simulink model "bee2_calib_corr"  (Simulink 7.1)
settings: Solver=ode45  StartTime=0.0  StopTime=100  RelTol=1e-3
undefined symbols (not defined in the file or in its folder): BEE2_usr
libraries: xbsIndex_r4 x2291, quant_xcorr_lib x1792, xps_library x56, xbsReplacements_r4 x32, casper_library_misc x2
(part 1 of 30)
settings Model: Creator=Administrator  LastModifiedBy=Jonathan Weintroub
settings DebuggingCC: SaveWithParameterizedLinksMsg=none  SignalResolutionControl=TryResolveAllWithWarning  MultiTaskDSMMsg=warning  MultiTaskCondExecSysMsg=none  StrictBusMsg=None
settings RTWCC: IncludeERTFirstTime=on
settings GRTTargetCC: TargetFcnLib=ansi_tfl_tmw.mat

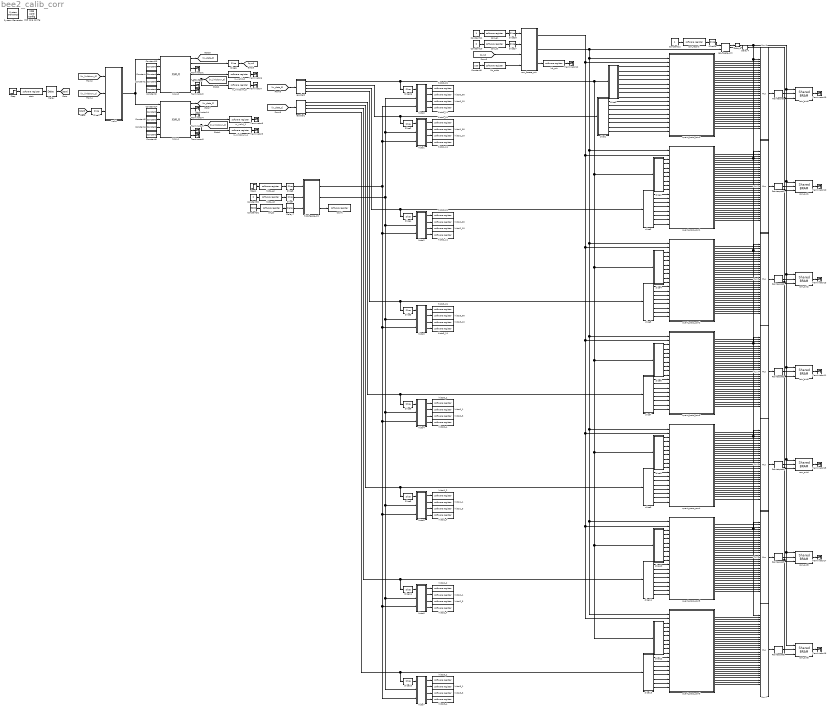
== system bee2_calib_corr ==
  " System Generator" : xbsIndex_r4/ System Generator  Tag=genX  SystemSampleTime=-1  xilinxfamily=virtex2p  part=xc2vp70  speed=-7  package=ff1704  synthesis_tool=XST  clock_wrapper=Clock Enables  directory=./bee2_calib_corr/sysgen  testbench=off  simulink_period=1  sysclk_period=10  dcm_input_clock_period=100  incr_netlist=off  trim_vbits=Everywhere in SubSystem  dbl_ovrd=According to Block Masks  core_generation=According to Block Masks  run_coregen=off  deprecated_control=off  eval_field=0  block_type=sysgen
      settings: block_version=10.1.3
  Constant : xbsIndex_r4/Constant  arith_type=Unsigned  const=0  n_bits=32  bin_pt=0  explicit_period=on  period=1  equ=P=C  opselect=C  inp2=PCIN>>17  opr=+  inp1=P  carry=CIN  dbl_ovrd=off  block_type=constant
  Constant1 : xbsIndex_r4/Constant  arith_type=Unsigned  const=0  n_bits=4  bin_pt=0  explicit_period=on  period=1  equ=P=C  opselect=C  inp2=PCIN>>17  opr=+  inp1=P  carry=CIN  dbl_ovrd=off  block_type=constant
  Constant10 : Constant  Value=0  OutDataType=sfix(16)  OutScaling=2^0
  Constant11 : Constant  OutDataType=sfix(16)  OutScaling=2^0
  Constant12 : Constant  OutDataType=sfix(16)  OutScaling=2^0
  Constant13 : xbsIndex_r4/Constant  arith_type=Boolean  const=0  n_bits=32  bin_pt=0  explicit_period=on  period=1  equ=P=C  opselect=C  inp2=PCIN>>17  opr=+  inp1=P  carry=CIN  dbl_ovrd=off  block_type=constant
  Constant14 : xbsIndex_r4/Constant  arith_type=Boolean  const=0  n_bits=32  bin_pt=0  explicit_period=on  period=1  equ=P=C  opselect=C  inp2=PCIN>>17  opr=+  inp1=P  carry=CIN  dbl_ovrd=off  block_type=constant
  Constant2 : xbsIndex_r4/Constant  arith_type=Boolean  const=0  n_bits=1  bin_pt=0  explicit_period=on  period=1  equ=P=C  opselect=C  inp2=PCIN>>17  opr=+  inp1=P  carry=CIN  dbl_ovrd=off  block_type=constant
  Constant3 : xbsIndex_r4/Constant  arith_type=Unsigned  const=0  n_bits=32  bin_pt=0  explicit_period=on  period=1  equ=P=C  opselect=C  inp2=PCIN>>17  opr=+  inp1=P  carry=CIN  dbl_ovrd=off  block_type=constant
  Constant31 : Constant  Value=5000  OutDataType=sfix(16)  OutScaling=2^0
  Constant32 : Constant  Value=0  OutDataType=sfix(16)  OutScaling=2^0
  Constant4 : xbsIndex_r4/Constant  arith_type=Unsigned  const=0  n_bits=4  bin_pt=0  explicit_period=on  period=1  equ=P=C  opselect=C  inp2=PCIN>>17  opr=+  inp1=P  carry=CIN  dbl_ovrd=off  block_type=constant
  Constant5 : xbsIndex_r4/Constant  arith_type=Boolean  const=0  n_bits=1  bin_pt=0  explicit_period=on  period=1  equ=P=C  opselect=C  inp2=PCIN>>17  opr=+  inp1=P  carry=CIN  dbl_ovrd=off  block_type=constant
  Constant8 : Constant  Value=100  OutDataType=sfix(16)  OutScaling=2^0
  Delay : xbsIndex_r4/Delay  en=off  latency=20  dbl_ovrd=off  reg_retiming=off  block_type=delay
      settings: xl_area=[0, 0, 0, 0, 0, 0, 0]
  Delay3 : xbsIndex_r4/Delay  en=off  latency=10  dbl_ovrd=off  reg_retiming=on  block_type=delay
      settings: xl_area=[0, 0, 0, 0, 0, 0, 0]
  Delay4 : xbsIndex_r4/Delay  en=off  latency=10  dbl_ovrd=off  reg_retiming=on  block_type=delay
      settings: xl_area=[0, 0, 0, 0, 0, 0, 0]
  Delay7 : xbsIndex_r4/Delay  en=off  latency=15  dbl_ovrd=off  reg_retiming=on  block_type=delay
      settings: xl_area=[0, 0, 0, 0, 0, 0, 0]
  Mux{1..7} (7 alike) : xbsIndex_r4/Mux  inputs=32  en=off  latency=0  precision=Full  arith_type="Signed  (2's comp)"  n_bits=8  bin_pt=2  quantization=Truncate  overflow=Wrap  dbl_ovrd=off  block_type=mux
      settings: xl_area=[0, 0, 0, 0, 0, 0, 0]
  Reinterpret{,1..6} (7 alike) : xbsIndex_r4/Reinterpret  force_arith_type=on  arith_type=Unsigned  force_bin_pt=on  bin_pt=0  block_type=reinterpret
      settings: infoedit="Changes signal type without altering the binary representation.   You can changed the signal between signed and unsigned, and relocate the binary point.<P><P>Hardware notes: In hardware this block costs nothing.<P><P>Example:  Suppose the input is 6 bits wide, signed, with 2 fractional bits, and the output is forced to unsigned with 0 fractional bits.  Then an input of -2.0 (1110.00 in binary 2's complement) becomes an output of 56 (111000 in binary)."  block_version=10.1.3
  Slice{1,6..8,27..29} (7 alike) : xbsIndex_r4/Slice  nbits=1  boolean_output=on  mode=Lower Bit Location + Width  bit1=0  base1=MSB of Input  bit0=0  base0=LSB of Input  dbl_ovrd=off  block_type=slice
      settings: infoedit="Extracts a given range of bits from each input sample and presents it at the output.  The output type is ordinarily unsigned with binary point at zero, but can be Boolean when the slice is one bit wide.<P><P>Hardware notes: In hardware this block costs nothing."  block_version=10.1.3
  Slice{2..5,9..11,26} (8 alike) : xbsIndex_r4/Slice  nbits=8  boolean_output=off  mode=Upper Bit Location + Width  bit1=0  base1=MSB of Input  bit0=0  base0=LSB of Input  dbl_ovrd=off  block_type=slice
      settings: infoedit="Extracts a given range of bits from each input sample and presents it at the output.  The output type is ordinarily unsigned with binary point at zero, but can be Boolean when the slice is one bit wide.<P><P>Hardware notes: In hardware this block costs nothing."  block_version=10.1.3
  Step : Step  Time=100  SampleTime=0
  Step1 : Step  Time=2000  SampleTime=0
  Terminator{,1..17,19} (19 alike) : Terminator
  XAUI_0 : xps_library/XAUI  Tag=xps:xaui  SystemSampleTime=-1  demux=1  port=BEE2_usr:0  open_phy=off  show_param=off  pre_emph=3  swing=800
      link override bee2_calib_corr_XAUI_0_rx_data: n_bits=64
      link override bee2_calib_corr_XAUI_0_rx_outofband: n_bits=8
      link override convert_tx_data: n_bits=64
      link override convert_tx_outofband: n_bits=8
  XAUI_1 : xps_library/XAUI  Tag=xps:xaui  SystemSampleTime=-1  demux=1  port=BEE2_usr:1  open_phy=off  show_param=off  pre_emph=3  swing=800
      link override bee2_calib_corr_XAUI_1_rx_data: n_bits=64
      link override bee2_calib_corr_XAUI_1_rx_outofband: n_bits=8
      link override convert_tx_data: n_bits=64
      link override convert_tx_outofband: n_bits=8
  XSG core config : xps_library/XSG core config  Tag=xps:xsg  SystemSampleTime=-1  hw_sys=BEE2_usr  ibob_linux=off  clk_src=sys_clk  iBOB_clk_src=sys_clk  BEE2_ctrl_clk_src=sys_clk  BEE2_usr_clk_src=sys_clk  ROACH_clk_src=sys_clk  CORR_clk_src=sys_clk  gpio_clk_io_group=iBOB:sma  gpio_clk_bit_index=0  clk_rate=100  sample_period=1  synthesis_tool=XST  mpc_type=powerpc405
  cnt_to : xps_library/software register  Tag=xps:sw_reg  SystemSampleTime=-1  io_dir=From Processor  arith_type=Unsigned  bitwidth=32  bin_pt=0  sample_period=1  gw_name=bee2_calib_corr_cnt_to_user_data_out
      link override bee2_calib_corr_cnt_to_user_data_out: arith_type=Unsigned  n_bits=32  bin_pt=0  period=1
  corr_en : xps_library/software register  Tag=xps:sw_reg  SystemSampleTime=-1  io_dir=From Processor  arith_type=Unsigned  bitwidth=32  bin_pt=0  sample_period=1  gw_name=bee2_calib_corr_corr_en_user_data_out
      link override bee2_calib_corr_corr_en_user_data_out: arith_type=Unsigned  n_bits=32  bin_pt=0  period=1
  corr_freeze_cnt : Subsystem
  corr_out0 : xps_library/Shared BRAM  Tag=xps:bram  SystemSampleTime=-1  arith_type="Signed  (2's comp)"  addr_width=11  data_width=32  data_bin_pt=0  init_vals=[1:2^11]  sample_rate=1
      link override bee2_calib_corr_corr_out0_data_out: arith_type="Signed  (2's comp)"
      link override convert_din: arith_type="Signed  (2's comp)"
      link override mem/sim_data_in: arith_type="Signed  (2's comp)"
  corr_out1 : xps_library/Shared BRAM  Tag=xps:bram  SystemSampleTime=-1  arith_type="Signed  (2's comp)"  addr_width=11  data_width=32  data_bin_pt=0  init_vals=[1:2^11]  sample_rate=1
      link override bee2_calib_corr_corr_out1_data_out: arith_type="Signed  (2's comp)"
      link override convert_din: arith_type="Signed  (2's comp)"
      link override mem/sim_data_in: arith_type="Signed  (2's comp)"
  corr_out2 : xps_library/Shared BRAM  Tag=xps:bram  SystemSampleTime=-1  arith_type="Signed  (2's comp)"  addr_width=11  data_width=32  data_bin_pt=0  init_vals=[1:2^11]  sample_rate=1
      link override bee2_calib_corr_corr_out2_data_out: arith_type="Signed  (2's comp)"
      link override convert_din: arith_type="Signed  (2's comp)"
      link override mem/sim_data_in: arith_type="Signed  (2's comp)"
  corr_out3 : xps_library/Shared BRAM  Tag=xps:bram  SystemSampleTime=-1  arith_type="Signed  (2's comp)"  addr_width=11  data_width=32  data_bin_pt=0  init_vals=[1:2^11]  sample_rate=1
      link override bee2_calib_corr_corr_out3_data_out: arith_type="Signed  (2's comp)"
      link override convert_din: arith_type="Signed  (2's comp)"
      link override mem/sim_data_in: arith_type="Signed  (2's comp)"
  corr_out4 : xps_library/Shared BRAM  Tag=xps:bram  SystemSampleTime=-1  arith_type="Signed  (2's comp)"  addr_width=11  data_width=32  data_bin_pt=0  init_vals=[1:2^11]  sample_rate=1
      link override bee2_calib_corr_corr_out4_data_out: arith_type="Signed  (2's comp)"
      link override convert_din: arith_type="Signed  (2's comp)"
      link override mem/sim_data_in: arith_type="Signed  (2's comp)"
  corr_out5 : xps_library/Shared BRAM  Tag=xps:bram  SystemSampleTime=-1  arith_type="Signed  (2's comp)"  addr_width=11  data_width=32  data_bin_pt=0  init_vals=[1:2^11]  sample_rate=1
      link override bee2_calib_corr_corr_out5_data_out: arith_type="Signed  (2's comp)"
      link override convert_din: arith_type="Signed  (2's comp)"
      link override mem/sim_data_in: arith_type="Signed  (2's comp)"
  corr_out6 : xps_library/Shared BRAM  Tag=xps:bram  SystemSampleTime=-1  arith_type="Signed  (2's comp)"  addr_width=11  data_width=32  data_bin_pt=0  init_vals=[1:2^11]  sample_rate=1
      link override bee2_calib_corr_corr_out6_data_out: arith_type="Signed  (2's comp)"
      link override convert_din: arith_type="Signed  (2's comp)"
      link override mem/sim_data_in: arith_type="Signed  (2's comp)"
  corr_record : xps_library/software register  Tag=xps:sw_reg  SystemSampleTime=-1  io_dir=From Processor  arith_type=Unsigned  bitwidth=32  bin_pt=0  sample_period=1  gw_name=bee2_calib_corr_corr_record_user_data_out
      link override bee2_calib_corr_corr_record_user_data_out: arith_type=Unsigned  n_bits=32  bin_pt=0  period=1
  corr_rst : xps_library/software register  Tag=xps:sw_reg  SystemSampleTime=-1  io_dir=From Processor  arith_type=Unsigned  bitwidth=32  bin_pt=0  sample_period=1  gw_name=bee2_calib_corr_corr_rst_user_data_out
      link override bee2_calib_corr_corr_rst_user_data_out: arith_type=Unsigned  n_bits=32  bin_pt=0  period=1
  count : xps_library/software register  Tag=xps:sw_reg  SystemSampleTime=-1  io_dir=To Processor  arith_type=Unsigned  bitwidth=32  bin_pt=0  sample_period=1  gw_name=bee2_calib_corr_count_user_data_in
      link override convert: arith_type=Unsigned  n_bits=32  bin_pt=0
  hb_cnt : xps_library/software register  Tag=xps:sw_reg  SystemSampleTime=-1  io_dir=To Processor  arith_type=Unsigned  bitwidth=32  bin_pt=0  sample_period=1  gw_name=bee2_calib_corr_hb_cnt_user_data_in
      link override convert: arith_type=Unsigned  n_bits=32  bin_pt=0
  hb_cntto : xps_library/software register  Tag=xps:sw_reg  SystemSampleTime=-1  io_dir=From Processor  arith_type=Unsigned  bitwidth=32  bin_pt=0  sample_period=1  gw_name=bee2_calib_corr_hb_cntto_user_data_out
      link override bee2_calib_corr_hb_cntto_user_data_out: arith_type=Unsigned  n_bits=32  bin_pt=0  period=1
  hist_freeze_cnt : Subsystem
  histo0 : Subsystem
  histo0_00 : xps_library/software register  Tag=xps:sw_reg  SystemSampleTime=-1  io_dir=To Processor  arith_type=Unsigned  bitwidth=32  bin_pt=0  sample_period=1  gw_name=bee2_calib_corr_histo0_00_user_data_in
      link override convert: arith_type=Unsigned  n_bits=32  bin_pt=0
  histo0_01 : xps_library/software register  Tag=xps:sw_reg  SystemSampleTime=-1  io_dir=To Processor  arith_type=Unsigned  bitwidth=32  bin_pt=0  sample_period=1  gw_name=bee2_calib_corr_histo0_01_user_data_in
      link override convert: arith_type=Unsigned  n_bits=32  bin_pt=0
  histo0_1 : xps_library/software register  Tag=xps:sw_reg  SystemSampleTime=-1  io_dir=To Processor  arith_type=Unsigned  bitwidth=32  bin_pt=0  sample_period=1  gw_name=bee2_calib_corr_histo0_1_user_data_in
      link override convert: arith_type=Unsigned  n_bits=32  bin_pt=0
  histo0_10 : xps_library/software register  Tag=xps:sw_reg  SystemSampleTime=-1  io_dir=To Processor  arith_type=Unsigned  bitwidth=32  bin_pt=0  sample_period=1  gw_name=bee2_calib_corr_histo0_10_user_data_in
      link override convert: arith_type=Unsigned  n_bits=32  bin_pt=0
  histo0_11 : xps_library/software register  Tag=xps:sw_reg  SystemSampleTime=-1  io_dir=To Processor  arith_type=Unsigned  bitwidth=32  bin_pt=0  sample_period=1  gw_name=bee2_calib_corr_histo0_11_user_data_in
      link override convert: arith_type=Unsigned  n_bits=32  bin_pt=0
  histo0_2 : xps_library/software register  Tag=xps:sw_reg  SystemSampleTime=-1  io_dir=To Processor  arith_type=Unsigned  bitwidth=32  bin_pt=0  sample_period=1  gw_name=bee2_calib_corr_histo0_2_user_data_in
      link override convert: arith_type=Unsigned  n_bits=32  bin_pt=0
  histo0_3 : xps_library/software register  Tag=xps:sw_reg  SystemSampleTime=-1  io_dir=To Processor  arith_type=Unsigned  bitwidth=32  bin_pt=0  sample_period=1  gw_name=bee2_calib_corr_histo0_3_user_data_in
      link override convert: arith_type=Unsigned  n_bits=32  bin_pt=0
  histo0_4 : xps_library/software register  Tag=xps:sw_reg  SystemSampleTime=-1  io_dir=To Processor  arith_type=Unsigned  bitwidth=32  bin_pt=0  sample_period=1  gw_name=bee2_calib_corr_histo0_4_user_data_in
      link override convert: arith_type=Unsigned  n_bits=32  bin_pt=0
  histo1 : Subsystem
  histo1_00 : xps_library/software register  Tag=xps:sw_reg  SystemSampleTime=-1  io_dir=To Processor  arith_type=Unsigned  bitwidth=32  bin_pt=0  sample_period=1  gw_name=bee2_calib_corr_histo1_00_user_data_in
      link override convert: arith_type=Unsigned  n_bits=32  bin_pt=0
  histo1_01 : xps_library/software register  Tag=xps:sw_reg  SystemSampleTime=-1  io_dir=To Processor  arith_type=Unsigned  bitwidth=32  bin_pt=0  sample_period=1  gw_name=bee2_calib_corr_histo1_01_user_data_in
      link override convert: arith_type=Unsigned  n_bits=32  bin_pt=0
  histo1_1 : xps_library/software register  Tag=xps:sw_reg  SystemSampleTime=-1  io_dir=To Processor  arith_type=Unsigned  bitwidth=32  bin_pt=0  sample_period=1  gw_name=bee2_calib_corr_histo1_1_user_data_in
      link override convert: arith_type=Unsigned  n_bits=32  bin_pt=0
  histo1_10 : xps_library/software register  Tag=xps:sw_reg  SystemSampleTime=-1  io_dir=To Processor  arith_type=Unsigned  bitwidth=32  bin_pt=0  sample_period=1  gw_name=bee2_calib_corr_histo1_10_user_data_in
      link override convert: arith_type=Unsigned  n_bits=32  bin_pt=0
  histo1_11 : xps_library/software register  Tag=xps:sw_reg  SystemSampleTime=-1  io_dir=To Processor  arith_type=Unsigned  bitwidth=32  bin_pt=0  sample_period=1  gw_name=bee2_calib_corr_histo1_11_user_data_in
      link override convert: arith_type=Unsigned  n_bits=32  bin_pt=0
  histo1_2 : xps_library/software register  Tag=xps:sw_reg  SystemSampleTime=-1  io_dir=To Processor  arith_type=Unsigned  bitwidth=32  bin_pt=0  sample_period=1  gw_name=bee2_calib_corr_histo1_2_user_data_in
      link override convert: arith_type=Unsigned  n_bits=32  bin_pt=0
  histo1_3 : xps_library/software register  Tag=xps:sw_reg  SystemSampleTime=-1  io_dir=To Processor  arith_type=Unsigned  bitwidth=32  bin_pt=0  sample_period=1  gw_name=bee2_calib_corr_histo1_3_user_data_in
      link override convert: arith_type=Unsigned  n_bits=32  bin_pt=0
  histo1_4 : xps_library/software register  Tag=xps:sw_reg  SystemSampleTime=-1  io_dir=To Processor  arith_type=Unsigned  bitwidth=32  bin_pt=0  sample_period=1  gw_name=bee2_calib_corr_histo1_4_user_data_in
      link override convert: arith_type=Unsigned  n_bits=32  bin_pt=0
  histo2 : Subsystem
  histo2_00 : xps_library/software register  Tag=xps:sw_reg  SystemSampleTime=-1  io_dir=To Processor  arith_type=Unsigned  bitwidth=32  bin_pt=0  sample_period=1  gw_name=bee2_calib_corr_histo2_00_user_data_in
      link override convert: arith_type=Unsigned  n_bits=32  bin_pt=0
  histo2_01 : xps_library/software register  Tag=xps:sw_reg  SystemSampleTime=-1  io_dir=To Processor  arith_type=Unsigned  bitwidth=32  bin_pt=0  sample_period=1  gw_name=bee2_calib_corr_histo2_01_user_data_in
      link override convert: arith_type=Unsigned  n_bits=32  bin_pt=0
  histo2_1 : xps_library/software register  Tag=xps:sw_reg  SystemSampleTime=-1  io_dir=To Processor  arith_type=Unsigned  bitwidth=32  bin_pt=0  sample_period=1  gw_name=bee2_calib_corr_histo2_1_user_data_in
      link override convert: arith_type=Unsigned  n_bits=32  bin_pt=0
  histo2_10 : xps_library/software register  Tag=xps:sw_reg  SystemSampleTime=-1  io_dir=To Processor  arith_type=Unsigned  bitwidth=32  bin_pt=0  sample_period=1  gw_name=bee2_calib_corr_histo2_10_user_data_in
      link override convert: arith_type=Unsigned  n_bits=32  bin_pt=0
  histo2_11 : xps_library/software register  Tag=xps:sw_reg  SystemSampleTime=-1  io_dir=To Processor  arith_type=Unsigned  bitwidth=32  bin_pt=0  sample_period=1  gw_name=bee2_calib_corr_histo2_11_user_data_in
      link override convert: arith_type=Unsigned  n_bits=32  bin_pt=0
  histo2_2 : xps_library/software register  Tag=xps:sw_reg  SystemSampleTime=-1  io_dir=To Processor  arith_type=Unsigned  bitwidth=32  bin_pt=0  sample_period=1  gw_name=bee2_calib_corr_histo2_2_user_data_in
      link override convert: arith_type=Unsigned  n_bits=32  bin_pt=0
  histo2_3 : xps_library/software register  Tag=xps:sw_reg  SystemSampleTime=-1  io_dir=To Processor  arith_type=Unsigned  bitwidth=32  bin_pt=0  sample_period=1  gw_name=bee2_calib_corr_histo2_3_user_data_in
      link override convert: arith_type=Unsigned  n_bits=32  bin_pt=0
  histo2_4 : xps_library/software register  Tag=xps:sw_reg  SystemSampleTime=-1  io_dir=To Processor  arith_type=Unsigned  bitwidth=32  bin_pt=0  sample_period=1  gw_name=bee2_calib_corr_histo2_4_user_data_in
      link override convert: arith_type=Unsigned  n_bits=32  bin_pt=0
  histo3 : Subsystem
  histo3_00 : xps_library/software register  Tag=xps:sw_reg  SystemSampleTime=-1  io_dir=To Processor  arith_type=Unsigned  bitwidth=32  bin_pt=0  sample_period=1  gw_name=bee2_calib_corr_histo3_00_user_data_in
      link override convert: arith_type=Unsigned  n_bits=32  bin_pt=0
  histo3_01 : xps_library/software register  Tag=xps:sw_reg  SystemSampleTime=-1  io_dir=To Processor  arith_type=Unsigned  bitwidth=32  bin_pt=0  sample_period=1  gw_name=bee2_calib_corr_histo3_01_user_data_in
      link override convert: arith_type=Unsigned  n_bits=32  bin_pt=0
  histo3_1 : xps_library/software register  Tag=xps:sw_reg  SystemSampleTime=-1  io_dir=To Processor  arith_type=Unsigned  bitwidth=32  bin_pt=0  sample_period=1  gw_name=bee2_calib_corr_histo3_1_user_data_in
      link override convert: arith_type=Unsigned  n_bits=32  bin_pt=0
  histo3_10 : xps_library/software register  Tag=xps:sw_reg  SystemSampleTime=-1  io_dir=To Processor  arith_type=Unsigned  bitwidth=32  bin_pt=0  sample_period=1  gw_name=bee2_calib_corr_histo3_10_user_data_in
      link override convert: arith_type=Unsigned  n_bits=32  bin_pt=0
  histo3_11 : xps_library/software register  Tag=xps:sw_reg  SystemSampleTime=-1  io_dir=To Processor  arith_type=Unsigned  bitwidth=32  bin_pt=0  sample_period=1  gw_name=bee2_calib_corr_histo3_11_user_data_in
      link override convert: arith_type=Unsigned  n_bits=32  bin_pt=0
  histo3_2 : xps_library/software register  Tag=xps:sw_reg  SystemSampleTime=-1  io_dir=To Processor  arith_type=Unsigned  bitwidth=32  bin_pt=0  sample_period=1  gw_name=bee2_calib_corr_histo3_2_user_data_in
      link override convert: arith_type=Unsigned  n_bits=32  bin_pt=0
  histo3_3 : xps_library/software register  Tag=xps:sw_reg  SystemSampleTime=-1  io_dir=To Processor  arith_type=Unsigned  bitwidth=32  bin_pt=0  sample_period=1  gw_name=bee2_calib_corr_histo3_3_user_data_in
      link override convert: arith_type=Unsigned  n_bits=32  bin_pt=0
  histo3_4 : xps_library/software register  Tag=xps:sw_reg  SystemSampleTime=-1  io_dir=To Processor  arith_type=Unsigned  bitwidth=32  bin_pt=0  sample_period=1  gw_name=bee2_calib_corr_histo3_4_user_data_in
      link override convert: arith_type=Unsigned  n_bits=32  bin_pt=0
  histo4 : Subsystem
  histo5 : Subsystem
  histo6 : Subsystem
  histo7 : Subsystem
  histo_en : xps_library/software register  Tag=xps:sw_reg  SystemSampleTime=-1  io_dir=From Processor  arith_type=Unsigned  bitwidth=32  bin_pt=0  sample_period=1  gw_name=bee2_calib_corr_histo_en_user_data_out
      link override bee2_calib_corr_histo_en_user_data_out: arith_type=Unsigned  n_bits=32  bin_pt=0  period=1
  histo_rst : xps_library/software register  Tag=xps:sw_reg  SystemSampleTime=-1  io_dir=From Processor  arith_type=Unsigned  bitwidth=32  bin_pt=0  sample_period=1  gw_name=bee2_calib_corr_histo_rst_user_data_out
      link override bee2_calib_corr_histo_rst_user_data_out: arith_type=Unsigned  n_bits=32  bin_pt=0  period=1
  quant_cross_corr0 : Subsystem  AncestorBlock=quant_xcorr_lib/quant_cross_corr  mask(quant_cross_corr){ants=2, demuxr2=3, bits=2, lags=4, range=positive and negative lags}
      mask prompts: ants "Antennas:"; demuxr2 "Demux (2^?):"; bits "Bitwidth:"; lags "Lags (in multiples of demux):"
      mask init:
      | quant_cross_corr_init(gcb,...
      |     'ants', ants,...
      |     'demuxr2', demuxr2,...
      |     'bits', bits,...
      |     'lags', lags,...
      |     'range', range);
      settings: AttributesFormatString=antennas=2, demux=8, lags=32
      mask StyleString: edit,edit,edit,edit,popup(positive and negative lags|positive lags only)
  quant_cross_corr1 : Subsystem  AncestorBlock=quant_xcorr_lib/quant_cross_corr  mask(quant_cross_corr){ants=2, demuxr2=3, bits=2, lags=4, range=positive and negative lags}
      mask prompts: ants "Antennas:"; demuxr2 "Demux (2^?):"; bits "Bitwidth:"; lags "Lags (in multiples of demux):"
      mask init:
      | quant_cross_corr_init(gcb,...
      |     'ants', ants,...
      |     'demuxr2', demuxr2,...
      |     'bits', bits,...
      |     'lags', lags,...
      |     'range', range);
      settings: AttributesFormatString=antennas=2, demux=8, lags=32
      mask StyleString: edit,edit,edit,edit,popup(positive and negative lags|positive lags only)
  quant_cross_corr2 : Subsystem  AncestorBlock=quant_xcorr_lib/quant_cross_corr  mask(quant_cross_corr){ants=2, demuxr2=3, bits=2, lags=4, range=positive and negative lags}
      mask prompts: ants "Antennas:"; demuxr2 "Demux (2^?):"; bits "Bitwidth:"; lags "Lags (in multiples of demux):"
      mask init:
      | quant_cross_corr_init(gcb,...
      |     'ants', ants,...
      |     'demuxr2', demuxr2,...
      |     'bits', bits,...
      |     'lags', lags,...
      |     'range', range);
      settings: AttributesFormatString=antennas=2, demux=8, lags=32
      mask StyleString: edit,edit,edit,edit,popup(positive and negative lags|positive lags only)
  quant_cross_corr3 : Subsystem  AncestorBlock=quant_xcorr_lib/quant_cross_corr  mask(quant_cross_corr){ants=2, demuxr2=3, bits=2, lags=4, range=positive and negative lags}
      mask prompts: ants "Antennas:"; demuxr2 "Demux (2^?):"; bits "Bitwidth:"; lags "Lags (in multiples of demux):"
      mask init:
      | quant_cross_corr_init(gcb,...
      |     'ants', ants,...
      |     'demuxr2', demuxr2,...
      |     'bits', bits,...
      |     'lags', lags,...
      |     'range', range);
      settings: AttributesFormatString=antennas=2, demux=8, lags=32
      mask StyleString: edit,edit,edit,edit,popup(positive and negative lags|positive lags only)
  quant_cross_corr4 : Subsystem  AncestorBlock=quant_xcorr_lib/quant_cross_corr  mask(quant_cross_corr){ants=2, demuxr2=3, bits=2, lags=4, range=positive and negative lags}
      mask prompts: ants "Antennas:"; demuxr2 "Demux (2^?):"; bits "Bitwidth:"; lags "Lags (in multiples of demux):"
      mask init:
      | quant_cross_corr_init(gcb,...
      |     'ants', ants,...
      |     'demuxr2', demuxr2,...
      |     'bits', bits,...
      |     'lags', lags,...
      |     'range', range);
      settings: AttributesFormatString=antennas=2, demux=8, lags=32
      mask StyleString: edit,edit,edit,edit,popup(positive and negative lags|positive lags only)
  quant_cross_corr5 : Subsystem  AncestorBlock=quant_xcorr_lib/quant_cross_corr  mask(quant_cross_corr){ants=2, demuxr2=3, bits=2, lags=4, range=positive and negative lags}
      mask prompts: ants "Antennas:"; demuxr2 "Demux (2^?):"; bits "Bitwidth:"; lags "Lags (in multiples of demux):"
      mask init:
      | quant_cross_corr_init(gcb,...
      |     'ants', ants,...
      |     'demuxr2', demuxr2,...
      |     'bits', bits,...
      |     'lags', lags,...
      |     'range', range);
      settings: AttributesFormatString=antennas=2, demux=8, lags=32
      mask StyleString: edit,edit,edit,edit,popup(positive and negative lags|positive lags only)
  quant_cross_corr6 : Subsystem  AncestorBlock=quant_xcorr_lib/quant_cross_corr  mask(quant_cross_corr){ants=2, demuxr2=3, bits=2, lags=4, range=positive and negative lags}
      mask prompts: ants "Antennas:"; demuxr2 "Demux (2^?):"; bits "Bitwidth:"; lags "Lags (in multiples of demux):"
      mask init:
      | quant_cross_corr_init(gcb,...
      |     'ants', ants,...
      |     'demuxr2', demuxr2,...
      |     'bits', bits,...
      |     'lags', lags,...
      |     'range', range);
      settings: AttributesFormatString=antennas=2, demux=8, lags=32
      mask StyleString: edit,edit,edit,edit,popup(positive and negative lags|positive lags only)
  rec_freeze_cnt : casper_library_misc/freeze_cntr  SystemSampleTime=-1  CounterBits=5
  reorder0 : Subsystem
  reorder1 : Subsystem
  rx_linkdown_0 : xps_library/software register  Tag=xps:sw_reg  SystemSampleTime=-1  io_dir=To Processor  arith_type=Unsigned  bitwidth=32  bin_pt=0  sample_period=1  gw_name=bee2_calib_corr_rx_linkdown_0_user_data_in
      link override convert: arith_type=Unsigned  n_bits=32  bin_pt=0
  rx_linkdown_1 : xps_library/software register  Tag=xps:sw_reg  SystemSampleTime=-1  io_dir=To Processor  arith_type=Unsigned  bitwidth=32  bin_pt=0  sample_period=1  gw_name=bee2_calib_corr_rx_linkdown_1_user_data_in
      link override convert: arith_type=Unsigned  n_bits=32  bin_pt=0
  rx_valid_0 : xps_library/software register  Tag=xps:sw_reg  SystemSampleTime=-1  io_dir=To Processor  arith_type=Unsigned  bitwidth=32  bin_pt=0  sample_period=1  gw_name=bee2_calib_corr_rx_valid_0_user_data_in
      link override convert: arith_type=Unsigned  n_bits=32  bin_pt=0
  rx_valid_1 : xps_library/software register  Tag=xps:sw_reg  SystemSampleTime=-1  io_dir=To Processor  arith_type=Unsigned  bitwidth=32  bin_pt=0  sample_period=1  gw_name=bee2_calib_corr_rx_valid_1_user_data_in
      link override convert: arith_type=Unsigned  n_bits=32  bin_pt=0
  slice0 : Subsystem
  slice1 : Subsystem
  slice10 : Subsystem
  slice11 : Subsystem
  slice12 : Subsystem
  slice13 : Subsystem
  slice2 : Subsystem
  slice3 : Subsystem
  slice4 : Subsystem
  slice5 : Subsystem
  slice6 : Subsystem
  slice7 : Subsystem
  slice8 : Subsystem
  slice9 : Subsystem
  start : xps_library/software register  Tag=xps:sw_reg  SystemSampleTime=-1  io_dir=From Processor  arith_type=Unsigned  bitwidth=32  bin_pt=0  sample_period=1  gw_name=bee2_calib_corr_start_user_data_out
      link override bee2_calib_corr_start_user_data_out: arith_type=Unsigned  n_bits=32  bin_pt=0  period=1
  valid : Subsystem
  wires:
  Step/1 -> start/1
  start/1 -> Delay/1
  Delay/1 -> [start]
  Constant/1 -> XAUI_0/3
  Constant1/1 -> XAUI_0/4
  Constant2/1 -> XAUI_0/5
  Constant3/1 -> XAUI_1/3
  Constant4/1 -> XAUI_1/4
  Constant5/1 -> XAUI_1/5
  XAUI_0/3 -> Terminator1/1
  XAUI_0/6 -> Terminator/1
  XAUI_0/7 -> Terminator2/1
  XAUI_1/3 -> Terminator4/1
  XAUI_1/6 -> Terminator3/1
  XAUI_1/7 -> Terminator5/1
  XAUI_0/4 -> rx_valid_0/1
  XAUI_0/5 -> rx_linkdown_0/1, [rx_linkdown_0]
  rx_valid_0/1 -> Terminator6/1
  rx_linkdown_0/1 -> Terminator7/1
  XAUI_1/4 -> rx_valid_1/1
  XAUI_1/5 -> [rx_linkdown_1], rx_linkdown_1/1
  rx_valid_1/1 -> Terminator8/1
  rx_linkdown_1/1 -> Terminator9/1
  [rx_linkdown_0] -> valid/1(ld0)
  [rx_linkdown_1] -> valid/2(ld1)
  XAUI_0/1 -> [rx_data_0]
  XAUI_1/1 -> [rx_data_1]
  [start] -> Slice1/1
  Slice1/1 -> valid/3(align)
  corr_freeze_cnt/2(rst_out) -> rec_freeze_cnt/2, quant_cross_corr0/1(rst), quant_cross_corr1/1(rst), quant_cross_corr2/1(rst), quant_cross_corr3/1(rst), quant_cross_corr6/1(rst), quant_cross_corr5/1(rst), quant_cross_corr4/1(rst)
  corr_freeze_cnt/1(en_out) -> quant_cross_corr0/2(en), quant_cross_corr1/2(en), quant_cross_corr2/2(en), quant_cross_corr3/2(en), quant_cross_corr6/2(en), quant_cross_corr5/2(en), quant_cross_corr4/2(en)
  corr_record/1 -> Slice29/1
  Constant12/1 -> corr_record/1
  Slice29/1 -> rec_freeze_cnt/1
  rec_freeze_cnt/1 -> Delay3/1
  Mux1/1 -> Reinterpret/1
  rec_freeze_cnt/2 -> Delay4/1
  corr_out0/1 -> Terminator19/1
  Reinterpret/1 -> corr_out0/2
  quant_cross_corr0/1(out-16) -> Mux1/2
  quant_cross_corr0/2(out-15) -> Mux1/3
  quant_cross_corr0/3(out-14) -> Mux1/4
  quant_cross_corr0/4(out-13) -> Mux1/5
  quant_cross_corr0/5(out-12) -> Mux1/6
  quant_cross_corr0/6(out-11) -> Mux1/7
  quant_cross_corr0/7(out-10) -> Mux1/8
  quant_cross_corr0/8(out-9) -> Mux1/9
  quant_cross_corr0/9(out-8) -> Mux1/10
  quant_cross_corr0/10(out-7) -> Mux1/11
  quant_cross_corr0/11(out-6) -> Mux1/12
  quant_cross_corr0/12(out-5) -> Mux1/13
  quant_cross_corr0/13(out-4) -> Mux1/14
  quant_cross_corr0/14(out-3) -> Mux1/15
  quant_cross_corr0/15(out-2) -> Mux1/16
  quant_cross_corr0/16(out-1) -> Mux1/17
  quant_cross_corr0/17(out0) -> Mux1/18
  quant_cross_corr0/18(out1) -> Mux1/19
  quant_cross_corr0/19(out2) -> Mux1/20
  quant_cross_corr0/20(out3) -> Mux1/21
  quant_cross_corr0/21(out4) -> Mux1/22
  quant_cross_corr0/22(out5) -> Mux1/23
  quant_cross_corr0/23(out6) -> Mux1/24
  quant_cross_corr0/24(out7) -> Mux1/25
  quant_cross_corr0/25(out8) -> Mux1/26
  quant_cross_corr0/26(out9) -> Mux1/27
  quant_cross_corr0/27(out10) -> Mux1/28
  quant_cross_corr0/28(out11) -> Mux1/29
  quant_cross_corr0/29(out12) -> Mux1/30
  quant_cross_corr0/30(out13) -> Mux1/31
  quant_cross_corr0/31(out14) -> Mux1/32
  quant_cross_corr0/32(out15) -> Mux1/33
  XAUI_0/2 -> Slice6/1
  XAUI_1/2 -> Terminator14/1
  valid/1(get) -> XAUI_0/1, XAUI_1/1
  Constant13/1 -> XAUI_0/2
  Constant14/1 -> XAUI_1/2
  [rx_data_0] -> reorder0/1(in)
  [rx_data_1] -> reorder1/1(in)
  slice0/1(out0) -> quant_cross_corr0/3(in1_16)
  slice0/2(out1) -> quant_cross_corr0/4(in1_17)
  slice0/3(out2) -> quant_cross_corr0/5(in1_18)
  slice0/4(out3) -> quant_cross_corr0/6(in1_19)
  slice0/5(out4) -> quant_cross_corr0/7(in1_20)
  slice0/6(out5) -> quant_cross_corr0/8(in1_21)
  slice0/7(out6) -> quant_cross_corr0/9(in1_22)
  slice0/8(out7) -> quant_cross_corr0/10(in1_23)
  slice1/1(out0) -> quant_cross_corr0/11(in2_16)
  slice1/2(out1) -> quant_cross_corr0/12(in2_17)
  slice1/3(out2) -> quant_cross_corr0/13(in2_18)
  slice1/4(out3) -> quant_cross_corr0/14(in2_19)
  slice1/5(out4) -> quant_cross_corr0/15(in2_20)
  slice1/6(out5) -> quant_cross_corr0/16(in2_21)
  slice1/7(out6) -> quant_cross_corr0/17(in2_22)
  slice1/8(out7) -> quant_cross_corr0/18(in2_23)
  Mux2/1 -> Reinterpret1/1
  corr_out1/1 -> Terminator10/1
  Reinterpret1/1 -> corr_out1/2
  quant_cross_corr1/8(out-9) -> Mux2/9
  quant_cross_corr1/9(out-8) -> Mux2/10
  quant_cross_corr1/10(out-7) -> Mux2/11
  quant_cross_corr1/11(out-6) -> Mux2/12
  quant_cross_corr1/12(out-5) -> Mux2/13
  quant_cross_corr1/13(out-4) -> Mux2/14
  quant_cross_corr1/14(out-3) -> Mux2/15
  quant_cross_corr1/15(out-2) -> Mux2/16
  quant_cross_corr1/16(out-1) -> Mux2/17
  quant_cross_corr1/17(out0) -> Mux2/18
  quant_cross_corr1/18(out1) -> Mux2/19
  quant_cross_corr1/19(out2) -> Mux2/20
  quant_cross_corr1/20(out3) -> Mux2/21
  quant_cross_corr1/21(out4) -> Mux2/22
  quant_cross_corr1/22(out5) -> Mux2/23
  quant_cross_corr1/23(out6) -> Mux2/24
  quant_cross_corr1/24(out7) -> Mux2/25
  quant_cross_corr1/25(out8) -> Mux2/26
  quant_cross_corr1/26(out9) -> Mux2/27
  quant_cross_corr1/27(out10) -> Mux2/28
  quant_cross_corr1/28(out11) -> Mux2/29
  quant_cross_corr1/29(out12) -> Mux2/30
  quant_cross_corr1/30(out13) -> Mux2/31
  quant_cross_corr1/31(out14) -> Mux2/32
  quant_cross_corr1/32(out15) -> Mux2/33
  slice2/3(out2) -> quant_cross_corr1/5(in1_18)
  slice2/4(out3) -> quant_cross_corr1/6(in1_19)
  slice2/5(out4) -> quant_cross_corr1/7(in1_20)
  slice2/6(out5) -> quant_cross_corr1/8(in1_21)
  slice2/7(out6) -> quant_cross_corr1/9(in1_22)
  slice2/8(out7) -> quant_cross_corr1/10(in1_23)
  slice3/1(out0) -> quant_cross_corr1/11(in2_16)
  slice3/2(out1) -> quant_cross_corr1/12(in2_17)
  slice3/3(out2) -> quant_cross_corr1/13(in2_18)
  slice3/4(out3) -> quant_cross_corr1/14(in2_19)
  slice3/5(out4) -> quant_cross_corr1/15(in2_20)
  slice3/6(out5) -> quant_cross_corr1/16(in2_21)
  slice3/7(out6) -> quant_cross_corr1/17(in2_22)
  slice3/8(out7) -> quant_cross_corr1/18(in2_23)
  quant_cross_corr1/1(out-16) -> Mux2/2
  quant_cross_corr1/2(out-15) -> Mux2/3
  quant_cross_corr1/3(out-14) -> Mux2/4
  quant_cross_corr1/4(out-13) -> Mux2/5
  quant_cross_corr1/5(out-12) -> Mux2/6
  quant_cross_corr1/6(out-11) -> Mux2/7
  quant_cross_corr1/7(out-10) -> Mux2/8
  reorder0/1(out0) -> Slice26/1, slice2/1(in), slice6/1(in), slice8/1(in), slice12/1(in), slice10/1(in), slice4/1(in), slice0/1(in)
  reorder0/2(out1) -> slice1/1(in), Slice2/1
  reorder0/3(out2) -> slice3/1(in), Slice4/1
  Mux3/1 -> Reinterpret2/1
  corr_out2/1 -> Terminator13/1
  Reinterpret2/1 -> corr_out2/2
  quant_cross_corr2/1(out-16) -> Mux3/2
  quant_cross_corr2/2(out-15) -> Mux3/3
  quant_cross_corr2/3(out-14) -> Mux3/4
  quant_cross_corr2/4(out-13) -> Mux3/5
  quant_cross_corr2/5(out-12) -> Mux3/6
  quant_cross_corr2/6(out-11) -> Mux3/7
  quant_cross_corr2/7(out-10) -> Mux3/8
  quant_cross_corr2/8(out-9) -> Mux3/9
  quant_cross_corr2/9(out-8) -> Mux3/10
  quant_cross_corr2/10(out-7) -> Mux3/11
  quant_cross_corr2/11(out-6) -> Mux3/12
  quant_cross_corr2/12(out-5) -> Mux3/13
  quant_cross_corr2/13(out-4) -> Mux3/14
  quant_cross_corr2/14(out-3) -> Mux3/15
  quant_cross_corr2/15(out-2) -> Mux3/16
  quant_cross_corr2/16(out-1) -> Mux3/17
  quant_cross_corr2/17(out0) -> Mux3/18
  quant_cross_corr2/18(out1) -> Mux3/19
  quant_cross_corr2/19(out2) -> Mux3/20
  quant_cross_corr2/20(out3) -> Mux3/21
  quant_cross_corr2/21(out4) -> Mux3/22
  quant_cross_corr2/22(out5) -> Mux3/23
  quant_cross_corr2/23(out6) -> Mux3/24
  quant_cross_corr2/24(out7) -> Mux3/25
  quant_cross_corr2/25(out8) -> Mux3/26
  quant_cross_corr2/26(out9) -> Mux3/27
  quant_cross_corr2/27(out10) -> Mux3/28
  quant_cross_corr2/28(out11) -> Mux3/29
  quant_cross_corr2/29(out12) -> Mux3/30
  quant_cross_corr2/30(out13) -> Mux3/31
  quant_cross_corr2/31(out14) -> Mux3/32
  quant_cross_corr2/32(out15) -> Mux3/33
  slice4/6(out5) -> quant_cross_corr2/8(in1_21)
  slice4/7(out6) -> quant_cross_corr2/9(in1_22)
  slice4/8(out7) -> quant_cross_corr2/10(in1_23)
  slice5/1(out0) -> quant_cross_corr2/11(in2_16)
  slice5/2(out1) -> quant_cross_corr2/12(in2_17)
  slice5/3(out2) -> quant_cross_corr2/13(in2_18)
  slice5/4(out3) -> quant_cross_corr2/14(in2_19)
  slice5/5(out4) -> quant_cross_corr2/15(in2_20)
  slice5/6(out5) -> quant_cross_corr2/16(in2_21)
  slice5/7(out6) -> quant_cross_corr2/17(in2_22)
  slice5/8(out7) -> quant_cross_corr2/18(in2_23)
  Mux4/1 -> Reinterpret3/1
  corr_out3/1 -> Terminator11/1
  Reinterpret3/1 -> corr_out3/2
  quant_cross_corr3/8(out-9) -> Mux4/9
  quant_cross_corr3/9(out-8) -> Mux4/10
  quant_cross_corr3/10(out-7) -> Mux4/11
  quant_cross_corr3/11(out-6) -> Mux4/12
  quant_cross_corr3/12(out-5) -> Mux4/13
  quant_cross_corr3/13(out-4) -> Mux4/14
  quant_cross_corr3/14(out-3) -> Mux4/15
  quant_cross_corr3/15(out-2) -> Mux4/16
  quant_cross_corr3/16(out-1) -> Mux4/17
  quant_cross_corr3/17(out0) -> Mux4/18
  quant_cross_corr3/18(out1) -> Mux4/19
  quant_cross_corr3/19(out2) -> Mux4/20
  quant_cross_corr3/20(out3) -> Mux4/21
  quant_cross_corr3/21(out4) -> Mux4/22
  quant_cross_corr3/22(out5) -> Mux4/23
  quant_cross_corr3/23(out6) -> Mux4/24
  quant_cross_corr3/24(out7) -> Mux4/25
  quant_cross_corr3/25(out8) -> Mux4/26
  quant_cross_corr3/26(out9) -> Mux4/27
  quant_cross_corr3/27(out10) -> Mux4/28
  quant_cross_corr3/28(out11) -> Mux4/29
  quant_cross_corr3/29(out12) -> Mux4/30
  quant_cross_corr3/30(out13) -> Mux4/31
  quant_cross_corr3/31(out14) -> Mux4/32
  quant_cross_corr3/32(out15) -> Mux4/33
  slice6/3(out2) -> quant_cross_corr3/5(in1_18)
  slice6/4(out3) -> quant_cross_corr3/6(in1_19)
  slice6/5(out4) -> quant_cross_corr3/7(in1_20)
  slice6/6(out5) -> quant_cross_corr3/8(in1_21)
  slice6/7(out6) -> quant_cross_corr3/9(in1_22)
  slice6/8(out7) -> quant_cross_corr3/10(in1_23)
  slice7/1(out0) -> quant_cross_corr3/11(in2_16)
  slice7/2(out1) -> quant_cross_corr3/12(in2_17)
  slice7/3(out2) -> quant_cross_corr3/13(in2_18)
  slice7/4(out3) -> quant_cross_corr3/14(in2_19)
  slice7/5(out4) -> quant_cross_corr3/15(in2_20)
  slice7/6(out5) -> quant_cross_corr3/16(in2_21)
  slice7/7(out6) -> quant_cross_corr3/17(in2_22)
  slice7/8(out7) -> quant_cross_corr3/18(in2_23)
  quant_cross_corr3/1(out-16) -> Mux4/2
  quant_cross_corr3/2(out-15) -> Mux4/3
  quant_cross_corr3/3(out-14) -> Mux4/4
  quant_cross_corr3/4(out-13) -> Mux4/5
  quant_cross_corr3/5(out-12) -> Mux4/6
  quant_cross_corr3/6(out-11) -> Mux4/7
  quant_cross_corr3/7(out-10) -> Mux4/8
  reorder0/4(out3) -> slice5/1(in), Slice3/1
  reorder1/1(out0) -> slice7/1(in), Slice9/1
  slice2/1(out0) -> quant_cross_corr1/3(in1_16)
  slice2/2(out1) -> quant_cross_corr1/4(in1_17)
  slice4/1(out0) -> quant_cross_corr2/3(in1_16)
  slice4/2(out1) -> quant_cross_corr2/4(in1_17)
  slice4/3(out2) -> quant_cross_corr2/5(in1_18)
  slice4/4(out3) -> quant_cross_corr2/6(in1_19)
  slice4/5(out4) -> quant_cross_corr2/7(in1_20)
  slice6/1(out0) -> quant_cross_corr3/3(in1_16)
  slice6/2(out1) -> quant_cross_corr3/4(in1_17)
  Mux5/1 -> Reinterpret4/1
  corr_out4/1 -> Terminator15/1
  Reinterpret4/1 -> corr_out4/2
  quant_cross_corr4/8(out-9) -> Mux5/9
  quant_cross_corr4/9(out-8) -> Mux5/10
  quant_cross_corr4/10(out-7) -> Mux5/11
  quant_cross_corr4/11(out-6) -> Mux5/12
  quant_cross_corr4/12(out-5) -> Mux5/13
  quant_cross_corr4/13(out-4) -> Mux5/14
  quant_cross_corr4/14(out-3) -> Mux5/15
  quant_cross_corr4/15(out-2) -> Mux5/16
  quant_cross_corr4/16(out-1) -> Mux5/17
  quant_cross_corr4/17(out0) -> Mux5/18
  quant_cross_corr4/18(out1) -> Mux5/19
  quant_cross_corr4/19(out2) -> Mux5/20
  quant_cross_corr4/20(out3) -> Mux5/21
  quant_cross_corr4/21(out4) -> Mux5/22
  quant_cross_corr4/22(out5) -> Mux5/23
  quant_cross_corr4/23(out6) -> Mux5/24
  quant_cross_corr4/24(out7) -> Mux5/25
  quant_cross_corr4/25(out8) -> Mux5/26
  quant_cross_corr4/26(out9) -> Mux5/27
  quant_cross_corr4/27(out10) -> Mux5/28
  quant_cross_corr4/28(out11) -> Mux5/29
  quant_cross_corr4/29(out12) -> Mux5/30
  quant_cross_corr4/30(out13) -> Mux5/31
  quant_cross_corr4/31(out14) -> Mux5/32
  quant_cross_corr4/32(out15) -> Mux5/33
  slice8/3(out2) -> quant_cross_corr4/5(in1_18)
  slice8/4(out3) -> quant_cross_corr4/6(in1_19)
  slice8/5(out4) -> quant_cross_corr4/7(in1_20)
  slice8/6(out5) -> quant_cross_corr4/8(in1_21)
  slice8/7(out6) -> quant_cross_corr4/9(in1_22)
  slice8/8(out7) -> quant_cross_corr4/10(in1_23)
  slice9/1(out0) -> quant_cross_corr4/11(in2_16)
  slice9/2(out1) -> quant_cross_corr4/12(in2_17)
  slice9/3(out2) -> quant_cross_corr4/13(in2_18)
  slice9/4(out3) -> quant_cross_corr4/14(in2_19)
  slice9/5(out4) -> quant_cross_corr4/15(in2_20)
  slice9/6(out5) -> quant_cross_corr4/16(in2_21)
  slice9/7(out6) -> quant_cross_corr4/17(in2_22)
  slice9/8(out7) -> quant_cross_corr4/18(in2_23)
  quant_cross_corr4/1(out-16) -> Mux5/2
  quant_cross_corr4/2(out-15) -> Mux5/3
  quant_cross_corr4/3(out-14) -> Mux5/4
  quant_cross_corr4/4(out-13) -> Mux5/5
  quant_cross_corr4/5(out-12) -> Mux5/6
  quant_cross_corr4/6(out-11) -> Mux5/7
  quant_cross_corr4/7(out-10) -> Mux5/8
  reorder1/2(out1) -> slice9/1(in), Slice5/1
  Mux6/1 -> Reinterpret5/1
  corr_out5/1 -> Terminator17/1
  Reinterpret5/1 -> corr_out5/2
  quant_cross_corr5/1(out-16) -> Mux6/2
  quant_cross_corr5/2(out-15) -> Mux6/3
  quant_cross_corr5/3(out-14) -> Mux6/4
  quant_cross_corr5/4(out-13) -> Mux6/5
  quant_cross_corr5/5(out-12) -> Mux6/6
  quant_cross_corr5/6(out-11) -> Mux6/7
  quant_cross_corr5/7(out-10) -> Mux6/8
  quant_cross_corr5/8(out-9) -> Mux6/9
  quant_cross_corr5/9(out-8) -> Mux6/10
  quant_cross_corr5/10(out-7) -> Mux6/11
  quant_cross_corr5/11(out-6) -> Mux6/12
  quant_cross_corr5/12(out-5) -> Mux6/13
  quant_cross_corr5/13(out-4) -> Mux6/14
  quant_cross_corr5/14(out-3) -> Mux6/15
  quant_cross_corr5/15(out-2) -> Mux6/16
  quant_cross_corr5/16(out-1) -> Mux6/17
  quant_cross_corr5/17(out0) -> Mux6/18
  quant_cross_corr5/18(out1) -> Mux6/19
  quant_cross_corr5/19(out2) -> Mux6/20
  quant_cross_corr5/20(out3) -> Mux6/21
  quant_cross_corr5/21(out4) -> Mux6/22
  quant_cross_corr5/22(out5) -> Mux6/23
  quant_cross_corr5/23(out6) -> Mux6/24
  quant_cross_corr5/24(out7) -> Mux6/25
  quant_cross_corr5/25(out8) -> Mux6/26
  quant_cross_corr5/26(out9) -> Mux6/27
  quant_cross_corr5/27(out10) -> Mux6/28
  quant_cross_corr5/28(out11) -> Mux6/29
  quant_cross_corr5/29(out12) -> Mux6/30
  quant_cross_corr5/30(out13) -> Mux6/31
  quant_cross_corr5/31(out14) -> Mux6/32
  quant_cross_corr5/32(out15) -> Mux6/33
  slice10/6(out5) -> quant_cross_corr5/8(in1_21)
  slice10/7(out6) -> quant_cross_corr5/9(in1_22)
  slice10/8(out7) -> quant_cross_corr5/10(in1_23)
  slice11/1(out0) -> quant_cross_corr5/11(in2_16)
  slice11/2(out1) -> quant_cross_corr5/12(in2_17)
  slice11/3(out2) -> quant_cross_corr5/13(in2_18)
  slice11/4(out3) -> quant_cross_corr5/14(in2_19)
  slice11/5(out4) -> quant_cross_corr5/15(in2_20)
  slice11/6(out5) -> quant_cross_corr5/16(in2_21)
  slice11/7(out6) -> quant_cross_corr5/17(in2_22)
  slice11/8(out7) -> quant_cross_corr5/18(in2_23)
  Mux7/1 -> Reinterpret6/1
  corr_out6/1 -> Terminator16/1
  Reinterpret6/1 -> corr_out6/2
  quant_cross_corr6/8(out-9) -> Mux7/9
  quant_cross_corr6/9(out-8) -> Mux7/10
  quant_cross_corr6/10(out-7) -> Mux7/11
  quant_cross_corr6/11(out-6) -> Mux7/12
  quant_cross_corr6/12(out-5) -> Mux7/13
  quant_cross_corr6/13(out-4) -> Mux7/14
  quant_cross_corr6/14(out-3) -> Mux7/15
  quant_cross_corr6/15(out-2) -> Mux7/16
  quant_cross_corr6/16(out-1) -> Mux7/17
  quant_cross_corr6/17(out0) -> Mux7/18
  quant_cross_corr6/18(out1) -> Mux7/19
  quant_cross_corr6/19(out2) -> Mux7/20
  quant_cross_corr6/20(out3) -> Mux7/21
  quant_cross_corr6/21(out4) -> Mux7/22
  quant_cross_corr6/22(out5) -> Mux7/23
  quant_cross_corr6/23(out6) -> Mux7/24
  quant_cross_corr6/24(out7) -> Mux7/25
  quant_cross_corr6/25(out8) -> Mux7/26
  quant_cross_corr6/26(out9) -> Mux7/27
  quant_cross_corr6/27(out10) -> Mux7/28
  quant_cross_corr6/28(out11) -> Mux7/29
  quant_cross_corr6/29(out12) -> Mux7/30
  quant_cross_corr6/30(out13) -> Mux7/31
  quant_cross_corr6/31(out14) -> Mux7/32
  quant_cross_corr6/32(out15) -> Mux7/33
  slice12/3(out2) -> quant_cross_corr6/5(in1_18)
  slice12/4(out3) -> quant_cross_corr6/6(in1_19)
  slice12/5(out4) -> quant_cross_corr6/7(in1_20)
  slice12/6(out5) -> quant_cross_corr6/8(in1_21)
  slice12/7(out6) -> quant_cross_corr6/9(in1_22)
  slice12/8(out7) -> quant_cross_corr6/10(in1_23)
  slice13/1(out0) -> quant_cross_corr6/11(in2_16)
  slice13/2(out1) -> quant_cross_corr6/12(in2_17)
  slice13/3(out2) -> quant_cross_corr6/13(in2_18)
  slice13/4(out3) -> quant_cross_corr6/14(in2_19)
  slice13/5(out4) -> quant_cross_corr6/15(in2_20)
  slice13/6(out5) -> quant_cross_corr6/16(in2_21)
  slice13/7(out6) -> quant_cross_corr6/17(in2_22)
  slice13/8(out7) -> quant_cross_corr6/18(in2_23)
  quant_cross_corr6/1(out-16) -> Mux7/2
  quant_cross_corr6/2(out-15) -> Mux7/3
  quant_cross_corr6/3(out-14) -> Mux7/4
  quant_cross_corr6/4(out-13) -> Mux7/5
  quant_cross_corr6/5(out-12) -> Mux7/6
  quant_cross_corr6/6(out-11) -> Mux7/7
  quant_cross_corr6/7(out-10) -> Mux7/8
  reorder1/3(out2) -> slice11/1(in), Slice11/1
  reorder1/4(out3) -> slice13/1(in), Slice10/1
  slice8/1(out0) -> quant_cross_corr4/3(in1_16)
  slice8/2(out1) -> quant_cross_corr4/4(in1_17)
  slice10/1(out0) -> quant_cross_corr5/3(in1_16)
  slice10/2(out1) -> quant_cross_corr5/4(in1_17)
  slice10/3(out2) -> quant_cross_corr5/5(in1_18)
  slice10/4(out3) -> quant_cross_corr5/6(in1_19)
  slice10/5(out4) -> quant_cross_corr5/7(in1_20)
  slice12/1(out0) -> quant_cross_corr6/3(in1_16)
  slice12/2(out1) -> quant_cross_corr6/4(in1_17)
  Constant10/1 -> corr_rst/1
  Constant11/1 -> corr_en/1
  corr_rst/1 -> Slice27/1
  corr_en/1 -> Slice28/1
  Slice28/1 -> corr_freeze_cnt/1(en)
  Slice27/1 -> corr_freeze_cnt/2(rst)
  Slice6/1 -> [sync]
  [sync] -> corr_freeze_cnt/3(sync)
  Constant8/1 -> hb_cntto/1
  hb_cntto/1 -> corr_freeze_cnt/4(cnt_to)
  hb_cnt/1 -> Terminator12/1
  corr_freeze_cnt/3(cnt) -> hb_cnt/1
  Delay3/1 -> Mux1/1, Mux2/1, Mux3/1, Mux4/1, Mux6/1, Mux7/1, Mux5/1, corr_out0/1, corr_out1/1, corr_out2/1, corr_out3/1, corr_out6/1, corr_out5/1, corr_out4/1
  Delay4/1 -> corr_out0/3, corr_out1/3, corr_out2/3, corr_out3/3, corr_out6/3, corr_out5/3, corr_out4/3
  Constant32/1 -> histo_rst/1
  Step1/1 -> histo_en/1
  histo_rst/1 -> Slice7/1
  histo_en/1 -> Slice8/1
  Slice8/1 -> hist_freeze_cnt/1(en)
  Slice7/1 -> hist_freeze_cnt/2(rst)
  hist_freeze_cnt/3(cnt) -> count/1
  Constant31/1 -> cnt_to/1
  cnt_to/1 -> Delay7/1
  Delay7/1 -> hist_freeze_cnt/3(cnt_to)
  histo0/1(out0) -> histo0_01/1
  histo0/2(out1) -> histo0_00/1
  histo0/3(out2) -> histo0_10/1
  histo0/4(out3) -> histo0_11/1
  histo1/1(out0) -> histo1_01/1
  histo1/2(out1) -> histo1_00/1
  histo1/3(out2) -> histo1_10/1
  histo1/4(out3) -> histo1_11/1
  histo2/1(out0) -> histo2_01/1
  histo2/2(out1) -> histo2_00/1
  histo2/3(out2) -> histo2_10/1
  histo2/4(out3) -> histo2_11/1
  Slice26/1 -> histo0/1(in)
  Slice2/1 -> histo1/1(in)
  Slice4/1 -> histo2/1(in)
  histo7/1(out0) -> histo3_2/1
  histo7/2(out1) -> histo3_1/1
  histo7/3(out2) -> histo3_3/1
  histo7/4(out3) -> histo3_4/1
  Slice10/1 -> histo7/1(in)
  histo6/1(out0) -> histo2_2/1
  Slice11/1 -> histo6/1(in)
  histo6/2(out1) -> histo2_1/1
  histo6/3(out2) -> histo2_3/1
  histo6/4(out3) -> histo2_4/1
  histo5/1(out0) -> histo1_2/1
  histo5/2(out1) -> histo1_1/1
  histo5/3(out2) -> histo1_3/1
  histo5/4(out3) -> histo1_4/1
  Slice5/1 -> histo5/1(in)
  histo4/1(out0) -> histo0_2/1
  histo4/2(out1) -> histo0_1/1
  histo4/3(out2) -> histo0_3/1
  histo4/4(out3) -> histo0_4/1
  Slice9/1 -> histo4/1(in)
  histo3/1(out0) -> histo3_01/1
  Slice3/1 -> histo3/1(in)
  histo3/2(out1) -> histo3_00/1
  histo3/3(out2) -> histo3_10/1
  histo3/4(out3) -> histo3_11/1
  hist_freeze_cnt/1(en_out) -> histo2/3(en), histo3/3(en), histo4/3(en), histo5/3(en), histo6/3(en), histo7/3(en), histo0/3(en), histo1/3(en)
  hist_freeze_cnt/2(rst_out) -> histo0/2(rst), histo1/2(rst), histo2/2(rst), histo3/2(rst), histo4/2(rst), histo5/2(rst), histo6/2(rst), histo7/2(rst)
